simulink model "cddDcmApp"  (R2022b)
settings: Solver=FixedStepDiscrete  StartTime=0.0  StopTime=10.0  FixedStep=0.02  RelTol=1e-3
undefined symbols (not defined in the file or in its folder): CCPFaultType, DcChlType, HFAChlType, IcuChannelType, MtrRoutStaEnum, P_SignalPartType, P_Std_ReturnType, PowerChlType, SrsAdChlType, VGAChlType
(part 7 of 13)

== system cddDcmApp/DataServices_DID_980E_DID_980E_1_Front_Blower_pwm/DataServices_DID_980E_DID_980E_1_Front_Blower_pwm_ShortTermAdjustment_sys ==
  function : TriggerPort  TriggerType=function-call  IsSimulinkFunction=on  FunctionName=DataServices_DID_980E_DID_980E_1_Front_Blower_pwm_ShortTermAdjustment  VariantControl=(inherit)
  Ground_1 : Ground
  Ground_2 : Ground
  Terminator_1 : Terminator
  Terminator_2 : Terminator
  ControlStateInfo : ArgIn  OutDataTypeStr=uint8  ArgumentName=ControlStateInfo
  OpStatus : ArgIn  Port=2  OutDataTypeStr=Enum: Dcm_OpStatusType  ArgumentName=OpStatus
  ErrorCode : ArgOut  OutDataTypeStr=Enum: Dcm_NegativeResponseCodeType  ArgumentName=ErrorCode
  ERR : ArgOut  Port=2  OutDataTypeStr=Std_ReturnType  ArgumentName=ERR
  wires:
  ControlStateInfo/1 -> Terminator_1/1
  OpStatus/1 -> Terminator_2/1
  Ground_1/1 -> ErrorCode/1
  Ground_2/1 -> ERR/1

== system cddDcmApp/DataServices_DID_980E_DID_980E_2_Front_Blower_speedLIN1/DataServices_DID_980E_DID_980E_2_Front_Blower_speedLIN1_FreezeCurrentState_sys ==
  function : TriggerPort  TriggerType=function-call  IsSimulinkFunction=on  FunctionName=DataServices_DID_980E_DID_980E_2_Front_Blower_speedLIN1_FreezeCurrentState  VariantControl=(inherit)
  Ground_1 : Ground
  Ground_2 : Ground
  Terminator_1 : Terminator
  OpStatus : ArgIn  OutDataTypeStr=Enum: Dcm_OpStatusType  ArgumentName=OpStatus
  ErrorCode : ArgOut  OutDataTypeStr=Enum: Dcm_NegativeResponseCodeType  ArgumentName=ErrorCode
  ERR : ArgOut  Port=2  OutDataTypeStr=Std_ReturnType  ArgumentName=ERR
  wires:
  OpStatus/1 -> Terminator_1/1
  Ground_1/1 -> ErrorCode/1
  Ground_2/1 -> ERR/1

== system cddDcmApp/DataServices_DID_980E_DID_980E_2_Front_Blower_speedLIN1/DataServices_DID_980E_DID_980E_2_Front_Blower_speedLIN1_ReadData_sys ==
  function : TriggerPort  TriggerType=function-call  IsSimulinkFunction=on  FunctionName=DataServices_DID_980E_DID_980E_2_Front_Blower_speedLIN1_ReadData  VariantControl=(inherit)
  Ground_1 : Ground
  Ground_2 : Ground
  Terminator_1 : Terminator
  OpStatus : ArgIn  OutDataTypeStr=Enum: Dcm_OpStatusType  ArgumentName=OpStatus
  Data : ArgOut  OutDataTypeStr=uint8  ArgumentName=Data
  ERR : ArgOut  Port=2  OutDataTypeStr=Std_ReturnType  ArgumentName=ERR
  wires:
  OpStatus/1 -> Terminator_1/1
  Ground_1/1 -> Data/1
  Ground_2/1 -> ERR/1

== system cddDcmApp/DataServices_DID_980E_DID_980E_2_Front_Blower_speedLIN1/DataServices_DID_980E_DID_980E_2_Front_Blower_speedLIN1_ReturnControlToECU_sys ==
  function : TriggerPort  TriggerType=function-call  IsSimulinkFunction=on  FunctionName=DataServices_DID_980E_DID_980E_2_Front_Blower_speedLIN1_ReturnControlToECU  VariantControl=(inherit)
  Ground_1 : Ground
  Ground_2 : Ground
  ErrorCode : ArgOut  OutDataTypeStr=Enum: Dcm_NegativeResponseCodeType  ArgumentName=ErrorCode
  ERR : ArgOut  Port=2  OutDataTypeStr=Std_ReturnType  ArgumentName=ERR
  wires:
  Ground_1/1 -> ErrorCode/1
  Ground_2/1 -> ERR/1

== system cddDcmApp/DataServices_DID_980E_DID_980E_2_Front_Blower_speedLIN1/DataServices_DID_980E_DID_980E_2_Front_Blower_speedLIN1_ShortTermAdjustment_sys ==
  function : TriggerPort  TriggerType=function-call  IsSimulinkFunction=on  FunctionName=DataServices_DID_980E_DID_980E_2_Front_Blower_speedLIN1_ShortTermAdjustment  VariantControl=(inherit)
  Ground_1 : Ground
  Ground_2 : Ground
  Terminator_1 : Terminator
  Terminator_2 : Terminator
  ControlStateInfo : ArgIn  OutDataTypeStr=uint8  ArgumentName=ControlStateInfo
  OpStatus : ArgIn  Port=2  OutDataTypeStr=Enum: Dcm_OpStatusType  ArgumentName=OpStatus
  ErrorCode : ArgOut  OutDataTypeStr=Enum: Dcm_NegativeResponseCodeType  ArgumentName=ErrorCode
  ERR : ArgOut  Port=2  OutDataTypeStr=Std_ReturnType  ArgumentName=ERR
  wires:
  ControlStateInfo/1 -> Terminator_1/1
  OpStatus/1 -> Terminator_2/1
  Ground_1/1 -> ErrorCode/1
  Ground_2/1 -> ERR/1

== system cddDcmApp/DataServices_DID_980E_DID_980E_3_Incar_motorActvCmd/DataServices_DID_980E_DID_980E_3_Incar_motorActvCmd_FreezeCurrentState_sys ==
  function : TriggerPort  TriggerType=function-call  IsSimulinkFunction=on  FunctionName=DataServices_DID_980E_DID_980E_3_Incar_motorActvCmd_FreezeCurrentState  VariantControl=(inherit)
  Ground_1 : Ground
  Ground_2 : Ground
  Terminator_1 : Terminator
  OpStatus : ArgIn  OutDataTypeStr=Enum: Dcm_OpStatusType  ArgumentName=OpStatus
  ErrorCode : ArgOut  OutDataTypeStr=Enum: Dcm_NegativeResponseCodeType  ArgumentName=ErrorCode
  ERR : ArgOut  Port=2  OutDataTypeStr=Std_ReturnType  ArgumentName=ERR
  wires:
  OpStatus/1 -> Terminator_1/1
  Ground_1/1 -> ErrorCode/1
  Ground_2/1 -> ERR/1

== system cddDcmApp/DataServices_DID_980E_DID_980E_3_Incar_motorActvCmd/DataServices_DID_980E_DID_980E_3_Incar_motorActvCmd_ReadData_sys ==
  function : TriggerPort  TriggerType=function-call  IsSimulinkFunction=on  FunctionName=DataServices_DID_980E_DID_980E_3_Incar_motorActvCmd_ReadData  VariantControl=(inherit)
  Ground_1 : Ground
  Ground_2 : Ground
  Terminator_1 : Terminator
  OpStatus : ArgIn  OutDataTypeStr=Enum: Dcm_OpStatusType  ArgumentName=OpStatus
  Data : ArgOut  OutDataTypeStr=uint8  ArgumentName=Data
  ERR : ArgOut  Port=2  OutDataTypeStr=Std_ReturnType  ArgumentName=ERR
  wires:
  OpStatus/1 -> Terminator_1/1
  Ground_1/1 -> Data/1
  Ground_2/1 -> ERR/1

== system cddDcmApp/DataServices_DID_980E_DID_980E_3_Incar_motorActvCmd/DataServices_DID_980E_DID_980E_3_Incar_motorActvCmd_ReturnControlToECU_sys ==
  function : TriggerPort  TriggerType=function-call  IsSimulinkFunction=on  FunctionName=DataServices_DID_980E_DID_980E_3_Incar_motorActvCmd_ReturnControlToECU  VariantControl=(inherit)
  Ground_1 : Ground
  Ground_2 : Ground
  ErrorCode : ArgOut  OutDataTypeStr=Enum: Dcm_NegativeResponseCodeType  ArgumentName=ErrorCode
  ERR : ArgOut  Port=2  OutDataTypeStr=Std_ReturnType  ArgumentName=ERR
  wires:
  Ground_1/1 -> ErrorCode/1
  Ground_2/1 -> ERR/1

== system cddDcmApp/DataServices_DID_980E_DID_980E_3_Incar_motorActvCmd/DataServices_DID_980E_DID_980E_3_Incar_motorActvCmd_ShortTermAdjustment_sys ==
  function : TriggerPort  TriggerType=function-call  IsSimulinkFunction=on  FunctionName=DataServices_DID_980E_DID_980E_3_Incar_motorActvCmd_ShortTermAdjustment  VariantControl=(inherit)
  Ground_1 : Ground
  Ground_2 : Ground
  Terminator_1 : Terminator
  Terminator_2 : Terminator
  ControlStateInfo : ArgIn  OutDataTypeStr=uint8  PortDimensions=2  ArgumentName=ControlStateInfo
  OpStatus : ArgIn  Port=2  OutDataTypeStr=Enum: Dcm_OpStatusType  ArgumentName=OpStatus
  ErrorCode : ArgOut  OutDataTypeStr=Enum: Dcm_NegativeResponseCodeType  ArgumentName=ErrorCode
  ERR : ArgOut  Port=2  OutDataTypeStr=Std_ReturnType  ArgumentName=ERR
  wires:
  ControlStateInfo/1 -> Terminator_1/1
  OpStatus/1 -> Terminator_2/1
  Ground_1/1 -> ErrorCode/1
  Ground_2/1 -> ERR/1

== system cddDcmApp/DataServices_DID_980E_DID_980E_4_Rear_blower_speed/DataServices_DID_980E_DID_980E_4_Rear_blower_speed_FreezeCurrentState_sys ==
  function : TriggerPort  TriggerType=function-call  IsSimulinkFunction=on  FunctionName=DataServices_DID_980E_DID_980E_4_Rear_blower_speed_FreezeCurrentState  VariantControl=(inherit)
  Ground_1 : Ground
  Ground_2 : Ground
  Terminator_1 : Terminator
  OpStatus : ArgIn  OutDataTypeStr=Enum: Dcm_OpStatusType  ArgumentName=OpStatus
  ErrorCode : ArgOut  OutDataTypeStr=Enum: Dcm_NegativeResponseCodeType  ArgumentName=ErrorCode
  ERR : ArgOut  Port=2  OutDataTypeStr=Std_ReturnType  ArgumentName=ERR
  wires:
  OpStatus/1 -> Terminator_1/1
  Ground_1/1 -> ErrorCode/1
  Ground_2/1 -> ERR/1

== system cddDcmApp/DataServices_DID_980E_DID_980E_4_Rear_blower_speed/DataServices_DID_980E_DID_980E_4_Rear_blower_speed_ReadData_sys ==
  function : TriggerPort  TriggerType=function-call  IsSimulinkFunction=on  FunctionName=DataServices_DID_980E_DID_980E_4_Rear_blower_speed_ReadData  VariantControl=(inherit)
  Ground_1 : Ground
  Ground_2 : Ground
  Terminator_1 : Terminator
  OpStatus : ArgIn  OutDataTypeStr=Enum: Dcm_OpStatusType  ArgumentName=OpStatus
  Data : ArgOut  OutDataTypeStr=uint8  ArgumentName=Data
  ERR : ArgOut  Port=2  OutDataTypeStr=Std_ReturnType  ArgumentName=ERR
  wires:
  OpStatus/1 -> Terminator_1/1
  Ground_1/1 -> Data/1
  Ground_2/1 -> ERR/1

== system cddDcmApp/DataServices_DID_980E_DID_980E_4_Rear_blower_speed/DataServices_DID_980E_DID_980E_4_Rear_blower_speed_ReturnControlToECU_sys ==
  function : TriggerPort  TriggerType=function-call  IsSimulinkFunction=on  FunctionName=DataServices_DID_980E_DID_980E_4_Rear_blower_speed_ReturnControlToECU  VariantControl=(inherit)
  Ground_1 : Ground
  Ground_2 : Ground
  ErrorCode : ArgOut  OutDataTypeStr=Enum: Dcm_NegativeResponseCodeType  ArgumentName=ErrorCode
  ERR : ArgOut  Port=2  OutDataTypeStr=Std_ReturnType  ArgumentName=ERR
  wires:
  Ground_1/1 -> ErrorCode/1
  Ground_2/1 -> ERR/1

== system cddDcmApp/DataServices_DID_980E_DID_980E_4_Rear_blower_speed/DataServices_DID_980E_DID_980E_4_Rear_blower_speed_ShortTermAdjustment_sys ==
  function : TriggerPort  TriggerType=function-call  IsSimulinkFunction=on  FunctionName=DataServices_DID_980E_DID_980E_4_Rear_blower_speed_ShortTermAdjustment  VariantControl=(inherit)
  Ground_1 : Ground
  Ground_2 : Ground
  Terminator_1 : Terminator
  Terminator_2 : Terminator
  ControlStateInfo : ArgIn  OutDataTypeStr=uint8  ArgumentName=ControlStateInfo
  OpStatus : ArgIn  Port=2  OutDataTypeStr=Enum: Dcm_OpStatusType  ArgumentName=OpStatus
  ErrorCode : ArgOut  OutDataTypeStr=Enum: Dcm_NegativeResponseCodeType  ArgumentName=ErrorCode
  ERR : ArgOut  Port=2  OutDataTypeStr=Std_ReturnType  ArgumentName=ERR
  wires:
  ControlStateInfo/1 -> Terminator_1/1
  OpStatus/1 -> Terminator_2/1
  Ground_1/1 -> ErrorCode/1
  Ground_2/1 -> ERR/1

== system cddDcmApp/DataServices_DID_980F_Data_Record/DataServices_DID_980F_Data_Record_FreezeCurrentState_sys ==
  function : TriggerPort  TriggerType=function-call  IsSimulinkFunction=on  FunctionName=DataServices_DID_980F_Data_Record_FreezeCurrentState  VariantControl=(inherit)
  Ground_1 : Ground
  Ground_2 : Ground
  Terminator_1 : Terminator
  OpStatus : ArgIn  OutDataTypeStr=Enum: Dcm_OpStatusType  ArgumentName=OpStatus
  ErrorCode : ArgOut  OutDataTypeStr=Enum: Dcm_NegativeResponseCodeType  ArgumentName=ErrorCode
  ERR : ArgOut  Port=2  OutDataTypeStr=Std_ReturnType  ArgumentName=ERR
  wires:
  OpStatus/1 -> Terminator_1/1
  Ground_1/1 -> ErrorCode/1
  Ground_2/1 -> ERR/1

== system cddDcmApp/DataServices_DID_980F_Data_Record/DataServices_DID_980F_Data_Record_ReadData_sys ==
  function : TriggerPort  TriggerType=function-call  IsSimulinkFunction=on  FunctionName=DataServices_DID_980F_Data_Record_ReadData  VariantControl=(inherit)
  Ground_1 : Ground
  Ground_2 : Ground
  Terminator_1 : Terminator
  OpStatus : ArgIn  OutDataTypeStr=Enum: Dcm_OpStatusType  ArgumentName=OpStatus
  Data : ArgOut  OutDataTypeStr=uint8  ArgumentName=Data
  ERR : ArgOut  Port=2  OutDataTypeStr=Std_ReturnType  ArgumentName=ERR
  wires:
  OpStatus/1 -> Terminator_1/1
  Ground_1/1 -> Data/1
  Ground_2/1 -> ERR/1

== system cddDcmApp/DataServices_DID_980F_Data_Record/DataServices_DID_980F_Data_Record_ReturnControlToECU_sys ==
  function : TriggerPort  TriggerType=function-call  IsSimulinkFunction=on  FunctionName=DataServices_DID_980F_Data_Record_ReturnControlToECU  VariantControl=(inherit)
  Ground_1 : Ground
  Ground_2 : Ground
  ErrorCode : ArgOut  OutDataTypeStr=Enum: Dcm_NegativeResponseCodeType  ArgumentName=ErrorCode
  ERR : ArgOut  Port=2  OutDataTypeStr=Std_ReturnType  ArgumentName=ERR
  wires:
  Ground_1/1 -> ErrorCode/1
  Ground_2/1 -> ERR/1

== system cddDcmApp/DataServices_DID_980F_Data_Record/DataServices_DID_980F_Data_Record_ShortTermAdjustment_sys ==
  function : TriggerPort  TriggerType=function-call  IsSimulinkFunction=on  FunctionName=DataServices_DID_980F_Data_Record_ShortTermAdjustment  VariantControl=(inherit)
  Ground_1 : Ground
  Ground_2 : Ground
  Terminator_1 : Terminator
  Terminator_2 : Terminator
  ControlStateInfo : ArgIn  OutDataTypeStr=uint8  ArgumentName=ControlStateInfo
  OpStatus : ArgIn  Port=2  OutDataTypeStr=Enum: Dcm_OpStatusType  ArgumentName=OpStatus
  ErrorCode : ArgOut  OutDataTypeStr=Enum: Dcm_NegativeResponseCodeType  ArgumentName=ErrorCode
  ERR : ArgOut  Port=2  OutDataTypeStr=Std_ReturnType  ArgumentName=ERR
  wires:
  ControlStateInfo/1 -> Terminator_1/1
  OpStatus/1 -> Terminator_2/1
  Ground_1/1 -> ErrorCode/1
  Ground_2/1 -> ERR/1

== system cddDcmApp/DataServices_DID_987B_DID_987B_HBMF_Failure/DataServices_DID_987B_DID_987B_HBMF_Failure_Reasons_sys ==
  function : TriggerPort  TriggerType=function-call  IsSimulinkFunction=on  FunctionName=DataServices_DID_987B_DID_987B_HBMF_Failure_Reasons  VariantControl=(inherit)
  Ground_1 : Ground
  Ground_2 : Ground
  Terminator_1 : Terminator
  OpStatus : ArgIn  OutDataTypeStr=Enum: Dcm_OpStatusType  ArgumentName=OpStatus
  Data : ArgOut  OutDataTypeStr=uint8  ArgumentName=Data
  ERR : ArgOut  Port=2  OutDataTypeStr=Std_ReturnType  ArgumentName=ERR
  wires:
  OpStatus/1 -> Terminator_1/1
  Ground_1/1 -> Data/1
  Ground_2/1 -> ERR/1

== system cddDcmApp/DataServices_DID_987C_DID_987C_HBMF_Feedback/DataServices_DID_987C_DID_987C_HBMF_Feedback_Datalog_sys ==
  function : TriggerPort  TriggerType=function-call  IsSimulinkFunction=on  FunctionName=DataServices_DID_987C_DID_987C_HBMF_Feedback_Datalog  VariantControl=(inherit)
  Ground_1 : Ground
  Ground_2 : Ground
  Terminator_1 : Terminator
  OpStatus : ArgIn  OutDataTypeStr=Enum: Dcm_OpStatusType  ArgumentName=OpStatus
  Data : ArgOut  OutDataTypeStr=uint8  ArgumentName=Data
  ERR : ArgOut  Port=2  OutDataTypeStr=Std_ReturnType  ArgumentName=ERR
  wires:
  OpStatus/1 -> Terminator_1/1
  Ground_1/1 -> Data/1
  Ground_2/1 -> ERR/1

== system cddDcmApp/DataServices_DID_987D_DID_987D_PMSI_Failure/DataServices_DID_987D_DID_987D_PMSI_Failure_Reasons_sys ==
  function : TriggerPort  TriggerType=function-call  IsSimulinkFunction=on  FunctionName=DataServices_DID_987D_DID_987D_PMSI_Failure_Reasons  VariantControl=(inherit)
  Ground_1 : Ground
  Ground_2 : Ground
  Terminator_1 : Terminator
  OpStatus : ArgIn  OutDataTypeStr=Enum: Dcm_OpStatusType  ArgumentName=OpStatus
  Data : ArgOut  OutDataTypeStr=uint8  ArgumentName=Data
  ERR : ArgOut  Port=2  OutDataTypeStr=Std_ReturnType  ArgumentName=ERR
  wires:
  OpStatus/1 -> Terminator_1/1
  Ground_1/1 -> Data/1
  Ground_2/1 -> ERR/1

== system cddDcmApp/DataServices_DID_987E_DID_987E_AFU_Failure/DataServices_DID_987E_DID_987E_AFU_Failure_Reasons_sys ==
  function : TriggerPort  TriggerType=function-call  IsSimulinkFunction=on  FunctionName=DataServices_DID_987E_DID_987E_AFU_Failure_Reasons  VariantControl=(inherit)
  Ground_1 : Ground
  Ground_2 : Ground
  Terminator_1 : Terminator
  OpStatus : ArgIn  OutDataTypeStr=Enum: Dcm_OpStatusType  ArgumentName=OpStatus
  Data : ArgOut  OutDataTypeStr=uint8  ArgumentName=Data
  ERR : ArgOut  Port=2  OutDataTypeStr=Std_ReturnType  ArgumentName=ERR
  wires:
  OpStatus/1 -> Terminator_1/1
  Ground_1/1 -> Data/1
  Ground_2/1 -> ERR/1

== system cddDcmApp/DataServices_DID_987F_DID_987F_CCM_Low_Voltage_Power_Limited/DataServices_DID_987F_DID_987F_CCM_Low_Voltage_Power_Limited_Infor_sys ==
  function : TriggerPort  TriggerType=function-call  IsSimulinkFunction=on  FunctionName=DataServices_DID_987F_DID_987F_CCM_Low_Voltage_Power_Limited_Infor  VariantControl=(inherit)
  Ground_1 : Ground
  Ground_2 : Ground
  Terminator_1 : Terminator
  OpStatus : ArgIn  OutDataTypeStr=Enum: Dcm_OpStatusType  ArgumentName=OpStatus
  Data : ArgOut  OutDataTypeStr=uint8  ArgumentName=Data
  ERR : ArgOut  Port=2  OutDataTypeStr=Std_ReturnType  ArgumentName=ERR
  wires:
  OpStatus/1 -> Terminator_1/1
  Ground_1/1 -> Data/1
  Ground_2/1 -> ERR/1

== system cddDcmApp/DataServices_DID_9880_DID_9880_PWM_Blower_Error_Internal_Info/DataServices_DID_9880_DID_9880_PWM_Blower_Error_Internal_Info_Datalog_sys ==
  function : TriggerPort  TriggerType=function-call  IsSimulinkFunction=on  FunctionName=DataServices_DID_9880_DID_9880_PWM_Blower_Error_Internal_Info_Datalog  VariantControl=(inherit)
  Ground_1 : Ground
  Ground_2 : Ground
  Terminator_1 : Terminator
  OpStatus : ArgIn  OutDataTypeStr=Enum: Dcm_OpStatusType  ArgumentName=OpStatus
  Data : ArgOut  OutDataTypeStr=uint8  ArgumentName=Data
  ERR : ArgOut  Port=2  OutDataTypeStr=Std_ReturnType  ArgumentName=ERR
  wires:
  OpStatus/1 -> Terminator_1/1
  Ground_1/1 -> Data/1
  Ground_2/1 -> ERR/1

== system cddDcmApp/DataServices_DID_988E_DID_988E_Front_PWM_Blower_Feedback/DataServices_DID_988E_DID_988E_Front_PWM_Blower_Feedback_Datalog_sys ==
  function : TriggerPort  TriggerType=function-call  IsSimulinkFunction=on  FunctionName=DataServices_DID_988E_DID_988E_Front_PWM_Blower_Feedback_Datalog  VariantControl=(inherit)
  Ground_1 : Ground
  Ground_2 : Ground
  Terminator_1 : Terminator
  OpStatus : ArgIn  OutDataTypeStr=Enum: Dcm_OpStatusType  ArgumentName=OpStatus
  Data : ArgOut  OutDataTypeStr=uint8  ArgumentName=Data
  ERR : ArgOut  Port=2  OutDataTypeStr=Std_ReturnType  ArgumentName=ERR
  wires:
  OpStatus/1 -> Terminator_1/1
  Ground_1/1 -> Data/1
  Ground_2/1 -> ERR/1

== system cddDcmApp/DataServices_DID_988F_DID_988F_Rear_Blower_Feedback/DataServices_DID_988F_DID_988F_Rear_Blower_Feedback_Voltage_sys ==
  function : TriggerPort  TriggerType=function-call  IsSimulinkFunction=on  FunctionName=DataServices_DID_988F_DID_988F_Rear_Blower_Feedback_Voltage  VariantControl=(inherit)
  Ground_1 : Ground
  Ground_2 : Ground
  Terminator_1 : Terminator
  OpStatus : ArgIn  OutDataTypeStr=Enum: Dcm_OpStatusType  ArgumentName=OpStatus
  Data : ArgOut  OutDataTypeStr=uint8  ArgumentName=Data
  ERR : ArgOut  Port=2  OutDataTypeStr=Std_ReturnType  ArgumentName=ERR
  wires:
  OpStatus/1 -> Terminator_1/1
  Ground_1/1 -> Data/1
  Ground_2/1 -> ERR/1

== system cddDcmApp/DataServices_DID_9890_DID_9890_VGA1_Failure/DataServices_DID_9890_DID_9890_VGA1_Failure_Reasons_sys ==
  function : TriggerPort  TriggerType=function-call  IsSimulinkFunction=on  FunctionName=DataServices_DID_9890_DID_9890_VGA1_Failure_Reasons  VariantControl=(inherit)
  Ground_1 : Ground
  Ground_2 : Ground
  Terminator_1 : Terminator
  OpStatus : ArgIn  OutDataTypeStr=Enum: Dcm_OpStatusType  ArgumentName=OpStatus
  Data : ArgOut  OutDataTypeStr=uint8  ArgumentName=Data
  ERR : ArgOut  Port=2  OutDataTypeStr=Std_ReturnType  ArgumentName=ERR
  wires:
  OpStatus/1 -> Terminator_1/1
  Ground_1/1 -> Data/1
  Ground_2/1 -> ERR/1

== system cddDcmApp/DataServices_DID_9891_DID_9891_VGA2_Failure/DataServices_DID_9891_DID_9891_VGA2_Failure_Reasons_sys ==
  function : TriggerPort  TriggerType=function-call  IsSimulinkFunction=on  FunctionName=DataServices_DID_9891_DID_9891_VGA2_Failure_Reasons  VariantControl=(inherit)
  Ground_1 : Ground
  Ground_2 : Ground
  Terminator_1 : Terminator
  OpStatus : ArgIn  OutDataTypeStr=Enum: Dcm_OpStatusType  ArgumentName=OpStatus
  Data : ArgOut  OutDataTypeStr=uint8  ArgumentName=Data
  ERR : ArgOut  Port=2  OutDataTypeStr=Std_ReturnType  ArgumentName=ERR
  wires:
  OpStatus/1 -> Terminator_1/1
  Ground_1/1 -> Data/1
  Ground_2/1 -> ERR/1

== system cddDcmApp/DataServices_DID_9892_DID_9892_VGA3_Failure/DataServices_DID_9892_DID_9892_VGA3_Failure_Reasons_sys ==
  function : TriggerPort  TriggerType=function-call  IsSimulinkFunction=on  FunctionName=DataServices_DID_9892_DID_9892_VGA3_Failure_Reasons  VariantControl=(inherit)
  Ground_1 : Ground
  Ground_2 : Ground
  Terminator_1 : Terminator
  OpStatus : ArgIn  OutDataTypeStr=Enum: Dcm_OpStatusType  ArgumentName=OpStatus
  Data : ArgOut  OutDataTypeStr=uint8  ArgumentName=Data
  ERR : ArgOut  Port=2  OutDataTypeStr=Std_ReturnType  ArgumentName=ERR
  wires:
  OpStatus/1 -> Terminator_1/1
  Ground_1/1 -> Data/1
  Ground_2/1 -> ERR/1

== system cddDcmApp/DataServices_DID_9893_DID_9893_VGA4_Failure/DataServices_DID_9893_DID_9893_VGA4_Failure_Reasons_sys ==
  function : TriggerPort  TriggerType=function-call  IsSimulinkFunction=on  FunctionName=DataServices_DID_9893_DID_9893_VGA4_Failure_Reasons  VariantControl=(inherit)
  Ground_1 : Ground
  Ground_2 : Ground
  Terminator_1 : Terminator
  OpStatus : ArgIn  OutDataTypeStr=Enum: Dcm_OpStatusType  ArgumentName=OpStatus
  Data : ArgOut  OutDataTypeStr=uint8  ArgumentName=Data
  ERR : ArgOut  Port=2  OutDataTypeStr=Std_ReturnType  ArgumentName=ERR
  wires:
  OpStatus/1 -> Terminator_1/1
  Ground_1/1 -> Data/1
  Ground_2/1 -> ERR/1

== system cddDcmApp/DataServices_DID_9894_DID_9894_VGA5_Failure/DataServices_DID_9894_DID_9894_VGA5_Failure_Reasons_sys ==
  function : TriggerPort  TriggerType=function-call  IsSimulinkFunction=on  FunctionName=DataServices_DID_9894_DID_9894_VGA5_Failure_Reasons  VariantControl=(inherit)
  Ground_1 : Ground
  Ground_2 : Ground
  Terminator_1 : Terminator
  OpStatus : ArgIn  OutDataTypeStr=Enum: Dcm_OpStatusType  ArgumentName=OpStatus
  Data : ArgOut  OutDataTypeStr=uint8  ArgumentName=Data
  ERR : ArgOut  Port=2  OutDataTypeStr=Std_ReturnType  ArgumentName=ERR
  wires:
  OpStatus/1 -> Terminator_1/1
  Ground_1/1 -> Data/1
  Ground_2/1 -> ERR/1

== system cddDcmApp/DataServices_DID_9895_DID_9895_VGA6_Failure/DataServices_DID_9895_DID_9895_VGA6_Failure_Reasons_sys ==
  function : TriggerPort  TriggerType=function-call  IsSimulinkFunction=on  FunctionName=DataServices_DID_9895_DID_9895_VGA6_Failure_Reasons  VariantControl=(inherit)
  Ground_1 : Ground
  Ground_2 : Ground
  Terminator_1 : Terminator
  OpStatus : ArgIn  OutDataTypeStr=Enum: Dcm_OpStatusType  ArgumentName=OpStatus
  Data : ArgOut  OutDataTypeStr=uint8  ArgumentName=Data
  ERR : ArgOut  Port=2  OutDataTypeStr=Std_ReturnType  ArgumentName=ERR
  wires:
  OpStatus/1 -> Terminator_1/1
  Ground_1/1 -> Data/1
  Ground_2/1 -> ERR/1

== system cddDcmApp/DataServices_DID_9896_DID_9896_VGA7_Failure/DataServices_DID_9896_DID_9896_VGA7_Failure_Reasons_sys ==
  function : TriggerPort  TriggerType=function-call  IsSimulinkFunction=on  FunctionName=DataServices_DID_9896_DID_9896_VGA7_Failure_Reasons  VariantControl=(inherit)
  Ground_1 : Ground
  Ground_2 : Ground
  Terminator_1 : Terminator
  OpStatus : ArgIn  OutDataTypeStr=Enum: Dcm_OpStatusType  ArgumentName=OpStatus
  Data : ArgOut  OutDataTypeStr=uint8  ArgumentName=Data
  ERR : ArgOut  Port=2  OutDataTypeStr=Std_ReturnType  ArgumentName=ERR
  wires:
  OpStatus/1 -> Terminator_1/1
  Ground_1/1 -> Data/1
  Ground_2/1 -> ERR/1

== system cddDcmApp/DataServices_DID_9897_DID_9897_VGA8_Failure/DataServices_DID_9897_DID_9897_VGA8_Failure_Reasons_sys ==
  function : TriggerPort  TriggerType=function-call  IsSimulinkFunction=on  FunctionName=DataServices_DID_9897_DID_9897_VGA8_Failure_Reasons  VariantControl=(inherit)
  Ground_1 : Ground
  Ground_2 : Ground
  Terminator_1 : Terminator
  OpStatus : ArgIn  OutDataTypeStr=Enum: Dcm_OpStatusType  ArgumentName=OpStatus
  Data : ArgOut  OutDataTypeStr=uint8  ArgumentName=Data
  ERR : ArgOut  Port=2  OutDataTypeStr=Std_ReturnType  ArgumentName=ERR
  wires:
  OpStatus/1 -> Terminator_1/1
  Ground_1/1 -> Data/1
  Ground_2/1 -> ERR/1

== system cddDcmApp/DataServices_DID_9898_DID_9898_Sensor_AD/DataServices_DID_9898_DID_9898_Sensor_AD_Value_sys ==
  function : TriggerPort  TriggerType=function-call  IsSimulinkFunction=on  FunctionName=DataServices_DID_9898_DID_9898_Sensor_AD_Value  VariantControl=(inherit)
  Ground_1 : Ground
  Ground_2 : Ground
  Terminator_1 : Terminator
  OpStatus : ArgIn  OutDataTypeStr=Enum: Dcm_OpStatusType  ArgumentName=OpStatus
  Data : ArgOut  OutDataTypeStr=uint8  ArgumentName=Data
  ERR : ArgOut  Port=2  OutDataTypeStr=Std_ReturnType  ArgumentName=ERR
  wires:
  OpStatus/1 -> Terminator_1/1
  Ground_1/1 -> Data/1
  Ground_2/1 -> ERR/1

== system cddDcmApp/DataServices_DID_989C_DID_989C_Rear_LIN_Blower_Module_VBMR_Fan_Status/DataServices_DID_989C_DID_989C_Rear_LIN_Blower_Module_VBMR_Fan_Status_ReadData_sys ==
  function : TriggerPort  TriggerType=function-call  IsSimulinkFunction=on  FunctionName=DataServices_DID_989C_DID_989C_Rear_LIN_Blower_Module_VBMR_Fan_Status_ReadData  VariantControl=(inherit)
  Ground_1 : Ground
  Ground_2 : Ground
  Terminator_1 : Terminator
  OpStatus : ArgIn  OutDataTypeStr=Enum: Dcm_OpStatusType  ArgumentName=OpStatus
  Data : ArgOut  OutDataTypeStr=uint8  ArgumentName=Data
  ERR : ArgOut  Port=2  OutDataTypeStr=Std_ReturnType  ArgumentName=ERR
  wires:
  OpStatus/1 -> Terminator_1/1
  Ground_1/1 -> Data/1
  Ground_2/1 -> ERR/1

== system cddDcmApp/DataServices_DID_989D_DID_989D_Rear_LIN_Blower_Module_VBMR_Feedback_Datalog/DataServices_DID_989D_DID_989D_Rear_LIN_Blower_Module_VBMR_Feedback_Datalog_ReadData_sys ==
  function : TriggerPort  TriggerType=function-call  IsSimulinkFunction=on  FunctionName=DataServices_DID_989D_DID_989D_Rear_LIN_Blower_Module_VBMR_Feedback_Datalog_ReadData  VariantControl=(inherit)
  Ground_1 : Ground
  Ground_2 : Ground
  Terminator_1 : Terminator
  OpStatus : ArgIn  OutDataTypeStr=Enum: Dcm_OpStatusType  ArgumentName=OpStatus
  Data : ArgOut  OutDataTypeStr=uint8  ArgumentName=Data
  ERR : ArgOut  Port=2  OutDataTypeStr=Std_ReturnType  ArgumentName=ERR
  wires:
  OpStatus/1 -> Terminator_1/1
  Ground_1/1 -> Data/1
  Ground_2/1 -> ERR/1

== system cddDcmApp/DataServices_DID_989E_DID_989E_Rear_HVAC_Flap_Actuator_RHFA_Failure_Reasons/DataServices_DID_989E_DID_989E_Rear_HVAC_Flap_Actuator_RHFA_Failure_Reasons_ReadData_sys ==
  function : TriggerPort  TriggerType=function-call  IsSimulinkFunction=on  FunctionName=DataServices_DID_989E_DID_989E_Rear_HVAC_Flap_Actuator_RHFA_Failure_Reasons_ReadData  VariantControl=(inherit)
  Ground_1 : Ground
  Ground_2 : Ground
  Terminator_1 : Terminator
  OpStatus : ArgIn  OutDataTypeStr=Enum: Dcm_OpStatusType  ArgumentName=OpStatus
  Data : ArgOut  OutDataTypeStr=uint8  ArgumentName=Data
  ERR : ArgOut  Port=2  OutDataTypeStr=Std_ReturnType  ArgumentName=ERR
  wires:
  OpStatus/1 -> Terminator_1/1
  Ground_1/1 -> Data/1
  Ground_2/1 -> ERR/1

== system cddDcmApp/DataServices_DID_989F_DID_989F_CCM_LIN_Actuator_Frame_Status/DataServices_DID_989F_DID_989F_CCM_LIN_Actuator_Frame_Status_ReadData_sys ==
  function : TriggerPort  TriggerType=function-call  IsSimulinkFunction=on  FunctionName=DataServices_DID_989F_DID_989F_CCM_LIN_Actuator_Frame_Status_ReadData  VariantControl=(inherit)
  Ground_1 : Ground
  Ground_2 : Ground
  Terminator_1 : Terminator
  OpStatus : ArgIn  OutDataTypeStr=Enum: Dcm_OpStatusType  ArgumentName=OpStatus
  Data : ArgOut  OutDataTypeStr=uint8  ArgumentName=Data
  ERR : ArgOut  Port=2  OutDataTypeStr=Std_ReturnType  ArgumentName=ERR
  wires:
  OpStatus/1 -> Terminator_1/1
  Ground_1/1 -> Data/1
  Ground_2/1 -> ERR/1

== system cddDcmApp/DataServices_DID_98A1_DID_98A1_98A1_DC_Motor_Feedback_Voltage/DataServices_DID_98A1_DID_98A1_98A1_DC_Motor_Feedback_Voltage_ReadData_sys ==
  function : TriggerPort  TriggerType=function-call  IsSimulinkFunction=on  FunctionName=DataServices_DID_98A1_DID_98A1_98A1_DC_Motor_Feedback_Voltage_ReadData  VariantControl=(inherit)
  Ground_1 : Ground
  Ground_2 : Ground
  Terminator_1 : Terminator
  OpStatus : ArgIn  OutDataTypeStr=Enum: Dcm_OpStatusType  ArgumentName=OpStatus
  Data : ArgOut  OutDataTypeStr=UInt8  ArgumentName=Data
  ERR : ArgOut  Port=2  OutDataTypeStr=Std_ReturnType  ArgumentName=ERR
  wires:
  OpStatus/1 -> Terminator_1/1
  Ground_1/1 -> Data/1
  Ground_2/1 -> ERR/1

== system cddDcmApp/DataServices_DID_98A2_DID_98A2_Front_Lpm_Blower_Feedback_Voltage_Difference/DataServices_DID_98A2_DID_98A2_Front_Lpm_Blower_Feedback_Voltage_Difference_ReadData_sys ==
  function : TriggerPort  TriggerType=function-call  IsSimulinkFunction=on  FunctionName=DataServices_DID_98A2_DID_98A2_Front_Lpm_Blower_Feedback_Voltage_Difference_ReadData  VariantControl=(inherit)
  Ground_1 : Ground
  Ground_2 : Ground
  Terminator_1 : Terminator
  OpStatus : ArgIn  OutDataTypeStr=Enum: Dcm_OpStatusType  ArgumentName=OpStatus
  Data : ArgOut  OutDataTypeStr=UInt8  ArgumentName=Data
  ERR : ArgOut  Port=2  OutDataTypeStr=Std_ReturnType  ArgumentName=ERR
  wires:
  OpStatus/1 -> Terminator_1/1
  Ground_1/1 -> Data/1
  Ground_2/1 -> ERR/1

== system cddDcmApp/DataServices_DID_98A3_DID_98A3_Advanced_Air_Cleaner_AAC_Feedback_Info/DataServices_DID_98A3_DID_98A3_Advanced_Air_Cleaner_AAC_Feedback_Info_ReadData_sys ==
  function : TriggerPort  TriggerType=function-call  IsSimulinkFunction=on  FunctionName=DataServices_DID_98A3_DID_98A3_Advanced_Air_Cleaner_AAC_Feedback_Info_ReadData  VariantControl=(inherit)
  Ground_1 : Ground
  Ground_2 : Ground
  Terminator_1 : Terminator
  OpStatus : ArgIn  OutDataTypeStr=Enum: Dcm_OpStatusType  ArgumentName=OpStatus
  Data : ArgOut  OutDataTypeStr=UInt8  ArgumentName=Data
  ERR : ArgOut  Port=2  OutDataTypeStr=Std_ReturnType  ArgumentName=ERR
  wires:
  OpStatus/1 -> Terminator_1/1
  Ground_1/1 -> Data/1
  Ground_2/1 -> ERR/1

== system cddDcmApp/DataServices_DID_98A4_DID_98A4_Oxygen_Generator_Module_OGM_Feedback_Info/DataServices_DID_98A4_DID_98A4_Oxygen_Generator_Module_OGM_Feedback_Info_ReadData_sys ==
  function : TriggerPort  TriggerType=function-call  IsSimulinkFunction=on  FunctionName=DataServices_DID_98A4_DID_98A4_Oxygen_Generator_Module_OGM_Feedback_Info_ReadData  VariantControl=(inherit)
  Ground_1 : Ground
  Ground_2 : Ground
  Terminator_1 : Terminator
  OpStatus : ArgIn  OutDataTypeStr=Enum: Dcm_OpStatusType  ArgumentName=OpStatus
  Data : ArgOut  OutDataTypeStr=UInt8  ArgumentName=Data
  ERR : ArgOut  Port=2  OutDataTypeStr=Std_ReturnType  ArgumentName=ERR
  wires:
  OpStatus/1 -> Terminator_1/1
  Ground_1/1 -> Data/1
  Ground_2/1 -> ERR/1

== system cddDcmApp/DataServices_DID_98A5_DID_98A5_Interior_Oxygen_Concentration_Sensor_IOSM_Module_Feedback_Info/DataServices_DID_98A5_DID_98A5_Interior_Oxygen_Concentration_Sensor_IOSM_Module_Feedback_Info_ReadData_sys ==
  function : TriggerPort  TriggerType=function-call  IsSimulinkFunction=on  FunctionName=DataServices_DID_98A5_DID_98A5_Interior_Oxygen_Concentration_Sensor_IOSM_Module_Feedback_Info_ReadData  VariantControl=(inherit)
  Ground_1 : Ground
  Ground_2 : Ground
  Terminator_1 : Terminator
  OpStatus : ArgIn  OutDataTypeStr=Enum: Dcm_OpStatusType  ArgumentName=OpStatus
  Data : ArgOut  OutDataTypeStr=UInt8  ArgumentName=Data
  ERR : ArgOut  Port=2  OutDataTypeStr=Std_ReturnType  ArgumentName=ERR
  wires:
  OpStatus/1 -> Terminator_1/1
  Ground_1/1 -> Data/1
  Ground_2/1 -> ERR/1

== system cddDcmApp/DataServices_DID_D910_DID_D910_BLW_THM_Output/DataServices_DID_D910_DID_D910_BLW_THM_Output_ReadData_sys ==
  function : TriggerPort  TriggerType=function-call  IsSimulinkFunction=on  FunctionName=DataServices_DID_D910_DID_D910_BLW_THM_Output_ReadData  VariantControl=(inherit)
  Ground_1 : Ground
  Ground_2 : Ground
  Data : ArgOut  OutDataTypeStr=uint8  ArgumentName=Data
  ERR : ArgOut  Port=2  OutDataTypeStr=Std_ReturnType  ArgumentName=ERR
  wires:
  Ground_1/1 -> Data/1
  Ground_2/1 -> ERR/1

== system cddDcmApp/DataServices_DID_D922_DID_D922_1_HVAC_Front_Blower_control_level/DataServices_DID_D922_DID_D922_1_HVAC_Front_Blower_control_level_ReadData_sys ==
  function : TriggerPort  TriggerType=function-call  IsSimulinkFunction=on  FunctionName=DataServices_DID_D922_DID_D922_1_HVAC_Front_Blower_control_level_ReadData  VariantControl=(inherit)
  Ground_1 : Ground
  Ground_2 : Ground
  Terminator_1 : Terminator
  OpStatus : ArgIn  OutDataTypeStr=Enum: Dcm_OpStatusType  ArgumentName=OpStatus
  Data : ArgOut  OutDataTypeStr=uint8  ArgumentName=Data
  ERR : ArgOut  Port=2  OutDataTypeStr=Std_ReturnType  ArgumentName=ERR
  wires:
  OpStatus/1 -> Terminator_1/1
  Ground_1/1 -> Data/1
  Ground_2/1 -> ERR/1

== system cddDcmApp/DataServices_DID_D922_DID_D922_2_HVAC_Rear_Blower_control_level/DataServices_DID_D922_DID_D922_2_HVAC_Rear_Blower_control_level_ReadData_sys ==
  function : TriggerPort  TriggerType=function-call  IsSimulinkFunction=on  FunctionName=DataServices_DID_D922_DID_D922_2_HVAC_Rear_Blower_control_level_ReadData  VariantControl=(inherit)
  Ground_1 : Ground
  Ground_2 : Ground
  Terminator_1 : Terminator
  OpStatus : ArgIn  OutDataTypeStr=Enum: Dcm_OpStatusType  ArgumentName=OpStatus
  Data : ArgOut  OutDataTypeStr=uint8  ArgumentName=Data
  ERR : ArgOut  Port=2  OutDataTypeStr=Std_ReturnType  ArgumentName=ERR
  wires:
  OpStatus/1 -> Terminator_1/1
  Ground_1/1 -> Data/1
  Ground_2/1 -> ERR/1

== system cddDcmApp/DataServices_DID_D924_DID_D924_1_No_Of_Movements/DataServices_DID_D924_DID_D924_1_No_Of_Movements_ReadData_sys ==
  function : TriggerPort  TriggerType=function-call  IsSimulinkFunction=on  FunctionName=DataServices_DID_D924_DID_D924_1_No_Of_Movements_ReadData  VariantControl=(inherit)
  Ground_1 : Ground
  Ground_2 : Ground
  Terminator_1 : Terminator
  OpStatus : ArgIn  OutDataTypeStr=Enum: Dcm_OpStatusType  ArgumentName=OpStatus
  Data : ArgOut  OutDataTypeStr=uint8  ArgumentName=Data
  ERR : ArgOut  Port=2  OutDataTypeStr=Std_ReturnType  ArgumentName=ERR
  wires:
  OpStatus/1 -> Terminator_1/1
  Ground_1/1 -> Data/1
  Ground_2/1 -> ERR/1

== system cddDcmApp/DataServices_DID_D924_DID_D924_1_No_Of_Movements/DataServices_DID_D924_DID_D924_1_No_Of_Movements_WriteData_sys ==
  function : TriggerPort  TriggerType=function-call  IsSimulinkFunction=on  FunctionName=DataServices_DID_D924_DID_D924_1_No_Of_Movements_WriteData  VariantControl=(inherit)
  Ground_1 : Ground
  Ground_2 : Ground
  Terminator_1 : Terminator
  Terminator_2 : Terminator
  Data : ArgIn  OutDataTypeStr=uint8  PortDimensions=4  ArgumentName=Data
  OpStatus : ArgIn  Port=2  OutDataTypeStr=Enum: Dcm_OpStatusType  ArgumentName=OpStatus
  ErrorCode : ArgOut  OutDataTypeStr=Enum: Dcm_NegativeResponseCodeType  ArgumentName=ErrorCode
  ERR : ArgOut  Port=2  OutDataTypeStr=Std_ReturnType  ArgumentName=ERR
  wires:
  Data/1 -> Terminator_1/1
  OpStatus/1 -> Terminator_2/1
  Ground_1/1 -> ErrorCode/1
  Ground_2/1 -> ERR/1

== system cddDcmApp/DataServices_DID_D924_DID_D924_2_ADC_value_Lower_Endstop_at_0/DataServices_DID_D924_DID_D924_2_ADC_value_Lower_Endstop_at_0_ReadData_sys ==
  function : TriggerPort  TriggerType=function-call  IsSimulinkFunction=on  FunctionName=DataServices_DID_D924_DID_D924_2_ADC_value_Lower_Endstop_at_0_ReadData  VariantControl=(inherit)
  Ground_1 : Ground
  Ground_2 : Ground
  Terminator_1 : Terminator
  OpStatus : ArgIn  OutDataTypeStr=Enum: Dcm_OpStatusType  ArgumentName=OpStatus
  Data : ArgOut  OutDataTypeStr=uint8  ArgumentName=Data
  ERR : ArgOut  Port=2  OutDataTypeStr=Std_ReturnType  ArgumentName=ERR
  wires:
  OpStatus/1 -> Terminator_1/1
  Ground_1/1 -> Data/1
  Ground_2/1 -> ERR/1

== system cddDcmApp/DataServices_DID_D924_DID_D924_2_ADC_value_Lower_Endstop_at_0/DataServices_DID_D924_DID_D924_2_ADC_value_Lower_Endstop_at_0_WriteData_sys ==
  function : TriggerPort  TriggerType=function-call  IsSimulinkFunction=on  FunctionName=DataServices_DID_D924_DID_D924_2_ADC_value_Lower_Endstop_at_0_WriteData  VariantControl=(inherit)
  Ground_1 : Ground
  Ground_2 : Ground
  Terminator_1 : Terminator
  Terminator_2 : Terminator
  Data : ArgIn  OutDataTypeStr=uint8  PortDimensions=2  ArgumentName=Data
  OpStatus : ArgIn  Port=2  OutDataTypeStr=Enum: Dcm_OpStatusType  ArgumentName=OpStatus
  ErrorCode : ArgOut  OutDataTypeStr=Enum: Dcm_NegativeResponseCodeType  ArgumentName=ErrorCode
  ERR : ArgOut  Port=2  OutDataTypeStr=Std_ReturnType  ArgumentName=ERR
  wires:
  Data/1 -> Terminator_1/1
  OpStatus/1 -> Terminator_2/1
  Ground_1/1 -> ErrorCode/1
  Ground_2/1 -> ERR/1

== system cddDcmApp/DataServices_DID_D924_DID_D924_3_ADC_value_Upper_Endstop_at_100/DataServices_DID_D924_DID_D924_3_ADC_value_Upper_Endstop_at_100_ReadData_sys ==
  function : TriggerPort  TriggerType=function-call  IsSimulinkFunction=on  FunctionName=DataServices_DID_D924_DID_D924_3_ADC_value_Upper_Endstop_at_100_ReadData  VariantControl=(inherit)
  Ground_1 : Ground
  Ground_2 : Ground
  Terminator_1 : Terminator
  OpStatus : ArgIn  OutDataTypeStr=Enum: Dcm_OpStatusType  ArgumentName=OpStatus
  Data : ArgOut  OutDataTypeStr=uint8  ArgumentName=Data
  ERR : ArgOut  Port=2  OutDataTypeStr=Std_ReturnType  ArgumentName=ERR
  wires:
  OpStatus/1 -> Terminator_1/1
  Ground_1/1 -> Data/1
  Ground_2/1 -> ERR/1

== system cddDcmApp/DataServices_DID_D924_DID_D924_3_ADC_value_Upper_Endstop_at_100/DataServices_DID_D924_DID_D924_3_ADC_value_Upper_Endstop_at_100_WriteData_sys ==
  function : TriggerPort  TriggerType=function-call  IsSimulinkFunction=on  FunctionName=DataServices_DID_D924_DID_D924_3_ADC_value_Upper_Endstop_at_100_WriteData  VariantControl=(inherit)
  Ground_1 : Ground
  Ground_2 : Ground
  Terminator_1 : Terminator
  Terminator_2 : Terminator
  Data : ArgIn  OutDataTypeStr=uint8  PortDimensions=2  ArgumentName=Data
  OpStatus : ArgIn  Port=2  OutDataTypeStr=Enum: Dcm_OpStatusType  ArgumentName=OpStatus
  ErrorCode : ArgOut  OutDataTypeStr=Enum: Dcm_NegativeResponseCodeType  ArgumentName=ErrorCode
  ERR : ArgOut  Port=2  OutDataTypeStr=Std_ReturnType  ArgumentName=ERR
  wires:
  Data/1 -> Terminator_1/1
  OpStatus/1 -> Terminator_2/1
  Ground_1/1 -> ErrorCode/1
  Ground_2/1 -> ERR/1

== system cddDcmApp/DataServices_DID_D925_ComplexStructure_2/DataServices_DID_D925_ComplexStructure_2_ReadData_sys ==
  function : TriggerPort  TriggerType=function-call  IsSimulinkFunction=on  FunctionName=DataServices_DID_D925_ComplexStructure_2_ReadData  VariantControl=(inherit)
  Ground_1 : Ground
  Ground_2 : Ground
  Terminator_1 : Terminator
  OpStatus : ArgIn  OutDataTypeStr=Enum: Dcm_OpStatusType  ArgumentName=OpStatus
  Data : ArgOut  OutDataTypeStr=uint8  ArgumentName=Data
  ERR : ArgOut  Port=2  OutDataTypeStr=Std_ReturnType  ArgumentName=ERR
  wires:
  OpStatus/1 -> Terminator_1/1
  Ground_1/1 -> Data/1
  Ground_2/1 -> ERR/1

== system cddDcmApp/DataServices_DID_D925_DID_D925_1_Requested_Position/DataServices_DID_D925_DID_D925_1_Requested_Position_ReadData_sys ==
  function : TriggerPort  TriggerType=function-call  IsSimulinkFunction=on  FunctionName=DataServices_DID_D925_DID_D925_1_Requested_Position_ReadData  VariantControl=(inherit)
  Ground_1 : Ground
  Ground_2 : Ground
  Terminator_1 : Terminator
  OpStatus : ArgIn  OutDataTypeStr=Enum: Dcm_OpStatusType  ArgumentName=OpStatus
  Data : ArgOut  OutDataTypeStr=uint8  ArgumentName=Data
  ERR : ArgOut  Port=2  OutDataTypeStr=Std_ReturnType  ArgumentName=ERR
  wires:
  OpStatus/1 -> Terminator_1/1
  Ground_1/1 -> Data/1
  Ground_2/1 -> ERR/1

== system cddDcmApp/DataServices_DID_D925_DID_D925_2_Actual_Position/DataServices_DID_D925_DID_D925_2_Actual_Position_ReadData_sys ==
  function : TriggerPort  TriggerType=function-call  IsSimulinkFunction=on  FunctionName=DataServices_DID_D925_DID_D925_2_Actual_Position_ReadData  VariantControl=(inherit)
  Ground_1 : Ground
  Ground_2 : Ground
  Terminator_1 : Terminator
  OpStatus : ArgIn  OutDataTypeStr=Enum: Dcm_OpStatusType  ArgumentName=OpStatus
  Data : ArgOut  OutDataTypeStr=uint8  ArgumentName=Data
  ERR : ArgOut  Port=2  OutDataTypeStr=Std_ReturnType  ArgumentName=ERR
  wires:
  OpStatus/1 -> Terminator_1/1
  Ground_1/1 -> Data/1
  Ground_2/1 -> ERR/1

== system cddDcmApp/DataServices_DID_D926_DID_D926_1_No_Of_Movements/DataServices_DID_D926_DID_D926_1_No_Of_Movements_ReadData_sys ==
  function : TriggerPort  TriggerType=function-call  IsSimulinkFunction=on  FunctionName=DataServices_DID_D926_DID_D926_1_No_Of_Movements_ReadData  VariantControl=(inherit)
  Ground_1 : Ground
  Ground_2 : Ground
  Terminator_1 : Terminator
  OpStatus : ArgIn  OutDataTypeStr=Enum: Dcm_OpStatusType  ArgumentName=OpStatus
  Data : ArgOut  OutDataTypeStr=uint8  ArgumentName=Data
  ERR : ArgOut  Port=2  OutDataTypeStr=Std_ReturnType  ArgumentName=ERR
  wires:
  OpStatus/1 -> Terminator_1/1
  Ground_1/1 -> Data/1
  Ground_2/1 -> ERR/1

== system cddDcmApp/DataServices_DID_D926_DID_D926_1_No_Of_Movements/DataServices_DID_D926_DID_D926_1_No_Of_Movements_WriteData_sys ==
  function : TriggerPort  TriggerType=function-call  IsSimulinkFunction=on  FunctionName=DataServices_DID_D926_DID_D926_1_No_Of_Movements_WriteData  VariantControl=(inherit)
  Ground_1 : Ground
  Ground_2 : Ground
  Terminator_1 : Terminator
  Terminator_2 : Terminator
  Data : ArgIn  OutDataTypeStr=uint8  PortDimensions=4  ArgumentName=Data
  OpStatus : ArgIn  Port=2  OutDataTypeStr=Enum: Dcm_OpStatusType  ArgumentName=OpStatus
  ErrorCode : ArgOut  OutDataTypeStr=Enum: Dcm_NegativeResponseCodeType  ArgumentName=ErrorCode
  ERR : ArgOut  Port=2  OutDataTypeStr=Std_ReturnType  ArgumentName=ERR
  wires:
  Data/1 -> Terminator_1/1
  OpStatus/1 -> Terminator_2/1
  Ground_1/1 -> ErrorCode/1
  Ground_2/1 -> ERR/1

== system cddDcmApp/DataServices_DID_D926_DID_D926_2_ADC_value_Lower_Endstop_at_0/DataServices_DID_D926_DID_D926_2_ADC_value_Lower_Endstop_at_0_ReadData_sys ==
  function : TriggerPort  TriggerType=function-call  IsSimulinkFunction=on  FunctionName=DataServices_DID_D926_DID_D926_2_ADC_value_Lower_Endstop_at_0_ReadData  VariantControl=(inherit)
  Ground_1 : Ground
  Ground_2 : Ground
  Terminator_1 : Terminator
  OpStatus : ArgIn  OutDataTypeStr=Enum: Dcm_OpStatusType  ArgumentName=OpStatus
  Data : ArgOut  OutDataTypeStr=uint8  ArgumentName=Data
  ERR : ArgOut  Port=2  OutDataTypeStr=Std_ReturnType  ArgumentName=ERR
  wires:
  OpStatus/1 -> Terminator_1/1
  Ground_1/1 -> Data/1
  Ground_2/1 -> ERR/1

== system cddDcmApp/DataServices_DID_D926_DID_D926_2_ADC_value_Lower_Endstop_at_0/DataServices_DID_D926_DID_D926_2_ADC_value_Lower_Endstop_at_0_WriteData_sys ==
  function : TriggerPort  TriggerType=function-call  IsSimulinkFunction=on  FunctionName=DataServices_DID_D926_DID_D926_2_ADC_value_Lower_Endstop_at_0_WriteData  VariantControl=(inherit)
  Ground_1 : Ground
  Ground_2 : Ground
  Terminator_1 : Terminator
  Terminator_2 : Terminator
  Data : ArgIn  OutDataTypeStr=uint8  PortDimensions=2  ArgumentName=Data
  OpStatus : ArgIn  Port=2  OutDataTypeStr=Enum: Dcm_OpStatusType  ArgumentName=OpStatus
  ErrorCode : ArgOut  OutDataTypeStr=Enum: Dcm_NegativeResponseCodeType  ArgumentName=ErrorCode
  ERR : ArgOut  Port=2  OutDataTypeStr=Std_ReturnType  ArgumentName=ERR
  wires:
  Data/1 -> Terminator_1/1
  OpStatus/1 -> Terminator_2/1
  Ground_1/1 -> ErrorCode/1
  Ground_2/1 -> ERR/1

== system cddDcmApp/DataServices_DID_D926_DID_D926_3_ADC_value_Upper_Endstop_at_100/DataServices_DID_D926_DID_D926_3_ADC_value_Upper_Endstop_at_100_ReadData_sys ==
  function : TriggerPort  TriggerType=function-call  IsSimulinkFunction=on  FunctionName=DataServices_DID_D926_DID_D926_3_ADC_value_Upper_Endstop_at_100_ReadData  VariantControl=(inherit)
  Ground_1 : Ground
  Ground_2 : Ground
  Terminator_1 : Terminator
  OpStatus : ArgIn  OutDataTypeStr=Enum: Dcm_OpStatusType  ArgumentName=OpStatus
  Data : ArgOut  OutDataTypeStr=uint8  ArgumentName=Data
  ERR : ArgOut  Port=2  OutDataTypeStr=Std_ReturnType  ArgumentName=ERR
  wires:
  OpStatus/1 -> Terminator_1/1
  Ground_1/1 -> Data/1
  Ground_2/1 -> ERR/1

== system cddDcmApp/DataServices_DID_D926_DID_D926_3_ADC_value_Upper_Endstop_at_100/DataServices_DID_D926_DID_D926_3_ADC_value_Upper_Endstop_at_100_WriteData_sys ==
  function : TriggerPort  TriggerType=function-call  IsSimulinkFunction=on  FunctionName=DataServices_DID_D926_DID_D926_3_ADC_value_Upper_Endstop_at_100_WriteData  VariantControl=(inherit)
  Ground_1 : Ground
  Ground_2 : Ground
  Terminator_1 : Terminator
  Terminator_2 : Terminator
  Data : ArgIn  OutDataTypeStr=uint8  PortDimensions=2  ArgumentName=Data
  OpStatus : ArgIn  Port=2  OutDataTypeStr=Enum: Dcm_OpStatusType  ArgumentName=OpStatus
  ErrorCode : ArgOut  OutDataTypeStr=Enum: Dcm_NegativeResponseCodeType  ArgumentName=ErrorCode
  ERR : ArgOut  Port=2  OutDataTypeStr=Std_ReturnType  ArgumentName=ERR
  wires:
  Data/1 -> Terminator_1/1
  OpStatus/1 -> Terminator_2/1
  Ground_1/1 -> ErrorCode/1
  Ground_2/1 -> ERR/1

== system cddDcmApp/DataServices_DID_D927_ComplexStructure_2/DataServices_DID_D927_ComplexStructure_2_ReadData_sys ==
  function : TriggerPort  TriggerType=function-call  IsSimulinkFunction=on  FunctionName=DataServices_DID_D927_ComplexStructure_2_ReadData  VariantControl=(inherit)
  Ground_1 : Ground
  Ground_2 : Ground
  Terminator_1 : Terminator
  OpStatus : ArgIn  OutDataTypeStr=Enum: Dcm_OpStatusType  ArgumentName=OpStatus
  Data : ArgOut  OutDataTypeStr=uint8  ArgumentName=Data
  ERR : ArgOut  Port=2  OutDataTypeStr=Std_ReturnType  ArgumentName=ERR
  wires:
  OpStatus/1 -> Terminator_1/1
  Ground_1/1 -> Data/1
  Ground_2/1 -> ERR/1

== system cddDcmApp/DataServices_DID_D927_DID_D927_1_Requested_Position/DataServices_DID_D927_DID_D927_1_Requested_Position_ReadData_sys ==
  function : TriggerPort  TriggerType=function-call  IsSimulinkFunction=on  FunctionName=DataServices_DID_D927_DID_D927_1_Requested_Position_ReadData  VariantControl=(inherit)
  Ground_1 : Ground
  Ground_2 : Ground
  Terminator_1 : Terminator
  OpStatus : ArgIn  OutDataTypeStr=Enum: Dcm_OpStatusType  ArgumentName=OpStatus
  Data : ArgOut  OutDataTypeStr=uint8  ArgumentName=Data
  ERR : ArgOut  Port=2  OutDataTypeStr=Std_ReturnType  ArgumentName=ERR
  wires:
  OpStatus/1 -> Terminator_1/1
  Ground_1/1 -> Data/1
  Ground_2/1 -> ERR/1

== system cddDcmApp/DataServices_DID_D927_DID_D927_2_Actual_Position/DataServices_DID_D927_DID_D927_2_Actual_Position_ReadData_sys ==
  function : TriggerPort  TriggerType=function-call  IsSimulinkFunction=on  FunctionName=DataServices_DID_D927_DID_D927_2_Actual_Position_ReadData  VariantControl=(inherit)
  Ground_1 : Ground
  Ground_2 : Ground
  Terminator_1 : Terminator
  OpStatus : ArgIn  OutDataTypeStr=Enum: Dcm_OpStatusType  ArgumentName=OpStatus
  Data : ArgOut  OutDataTypeStr=uint8  ArgumentName=Data
  ERR : ArgOut  Port=2  OutDataTypeStr=Std_ReturnType  ArgumentName=ERR
  wires:
  OpStatus/1 -> Terminator_1/1
  Ground_1/1 -> Data/1
  Ground_2/1 -> ERR/1

== system cddDcmApp/DataServices_DID_D928_DID_D928_1_No_Of_Movements/DataServices_DID_D928_DID_D928_1_No_Of_Movements_ReadData_sys ==
  function : TriggerPort  TriggerType=function-call  IsSimulinkFunction=on  FunctionName=DataServices_DID_D928_DID_D928_1_No_Of_Movements_ReadData  VariantControl=(inherit)
  Ground_1 : Ground
  Ground_2 : Ground
  Terminator_1 : Terminator
  OpStatus : ArgIn  OutDataTypeStr=Enum: Dcm_OpStatusType  ArgumentName=OpStatus
  Data : ArgOut  OutDataTypeStr=uint8  ArgumentName=Data
  ERR : ArgOut  Port=2  OutDataTypeStr=Std_ReturnType  ArgumentName=ERR
  wires:
  OpStatus/1 -> Terminator_1/1
  Ground_1/1 -> Data/1
  Ground_2/1 -> ERR/1

== system cddDcmApp/DataServices_DID_D928_DID_D928_1_No_Of_Movements/DataServices_DID_D928_DID_D928_1_No_Of_Movements_WriteData_sys ==
  function : TriggerPort  TriggerType=function-call  IsSimulinkFunction=on  FunctionName=DataServices_DID_D928_DID_D928_1_No_Of_Movements_WriteData  VariantControl=(inherit)
  Ground_1 : Ground
  Ground_2 : Ground
  Terminator_1 : Terminator
  Terminator_2 : Terminator
  Data : ArgIn  OutDataTypeStr=uint8  PortDimensions=4  ArgumentName=Data
  OpStatus : ArgIn  Port=2  OutDataTypeStr=Enum: Dcm_OpStatusType  ArgumentName=OpStatus
  ErrorCode : ArgOut  OutDataTypeStr=Enum: Dcm_NegativeResponseCodeType  ArgumentName=ErrorCode
  ERR : ArgOut  Port=2  OutDataTypeStr=Std_ReturnType  ArgumentName=ERR
  wires:
  Data/1 -> Terminator_1/1
  OpStatus/1 -> Terminator_2/1
  Ground_1/1 -> ErrorCode/1
  Ground_2/1 -> ERR/1

== system cddDcmApp/DataServices_DID_D928_DID_D928_2_ADC_value_Lower_Endstop_at_0/DataServices_DID_D928_DID_D928_2_ADC_value_Lower_Endstop_at_0_ReadData_sys ==
  function : TriggerPort  TriggerType=function-call  IsSimulinkFunction=on  FunctionName=DataServices_DID_D928_DID_D928_2_ADC_value_Lower_Endstop_at_0_ReadData  VariantControl=(inherit)
  Ground_1 : Ground
  Ground_2 : Ground
  Terminator_1 : Terminator
  OpStatus : ArgIn  OutDataTypeStr=Enum: Dcm_OpStatusType  ArgumentName=OpStatus
  Data : ArgOut  OutDataTypeStr=uint8  ArgumentName=Data
  ERR : ArgOut  Port=2  OutDataTypeStr=Std_ReturnType  ArgumentName=ERR
  wires:
  OpStatus/1 -> Terminator_1/1
  Ground_1/1 -> Data/1
  Ground_2/1 -> ERR/1

== system cddDcmApp/DataServices_DID_D928_DID_D928_2_ADC_value_Lower_Endstop_at_0/DataServices_DID_D928_DID_D928_2_ADC_value_Lower_Endstop_at_0_WriteData_sys ==
  function : TriggerPort  TriggerType=function-call  IsSimulinkFunction=on  FunctionName=DataServices_DID_D928_DID_D928_2_ADC_value_Lower_Endstop_at_0_WriteData  VariantControl=(inherit)
  Ground_1 : Ground
  Ground_2 : Ground
  Terminator_1 : Terminator
  Terminator_2 : Terminator
  Data : ArgIn  OutDataTypeStr=uint8  PortDimensions=2  ArgumentName=Data
  OpStatus : ArgIn  Port=2  OutDataTypeStr=Enum: Dcm_OpStatusType  ArgumentName=OpStatus
  ErrorCode : ArgOut  OutDataTypeStr=Enum: Dcm_NegativeResponseCodeType  ArgumentName=ErrorCode
  ERR : ArgOut  Port=2  OutDataTypeStr=Std_ReturnType  ArgumentName=ERR
  wires:
  Data/1 -> Terminator_1/1
  OpStatus/1 -> Terminator_2/1
  Ground_1/1 -> ErrorCode/1
  Ground_2/1 -> ERR/1
